simulink model "CCMDcMotor"  (R2022b) (continued)
== system CCMDcMotor/DcMotorIOCtrlService == (continued)
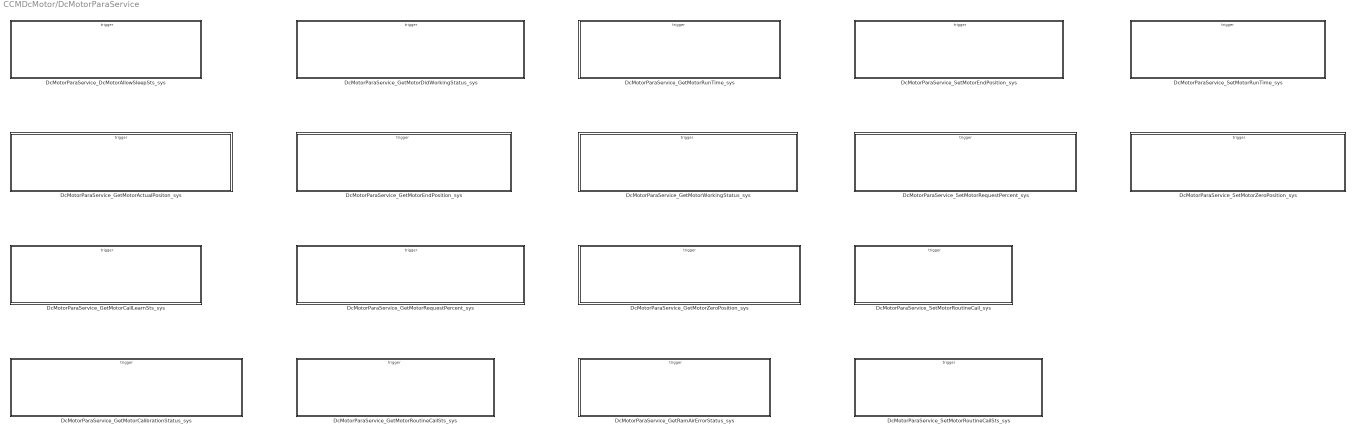
== system CCMDcMotor/DcMotorParaService ==
  DcMotorParaService_DcMotorAllowSleepSts_sys : Subsystem
  DcMotorParaService_GetMotorActualPositon_sys : Subsystem
  DcMotorParaService_GetMotorCailLearnSts_sys : Subsystem
  DcMotorParaService_GetMotorCalibrationStatus_sys : Subsystem
  DcMotorParaService_GetMotorDidWorkingStatus_sys : Subsystem
  DcMotorParaService_GetMotorEndPosition_sys : Subsystem
  DcMotorParaService_GetMotorRequestPercent_sys : Subsystem
  DcMotorParaService_GetMotorRoutineCailSts_sys : Subsystem
  DcMotorParaService_GetMotorRunTime_sys : Subsystem
  DcMotorParaService_GetMotorWorkingStatus_sys : Subsystem
  DcMotorParaService_GetMotorZeroPosition_sys : Subsystem
  DcMotorParaService_GetRamAirErrorStatus_sys : Subsystem
  DcMotorParaService_SetMotorEndPosition_sys : Subsystem
  DcMotorParaService_SetMotorRequestPercent_sys : Subsystem
  DcMotorParaService_SetMotorRoutineCailSts_sys : Subsystem
  DcMotorParaService_SetMotorRoutineCail_sys : Subsystem
  DcMotorParaService_SetMotorRunTime_sys : Subsystem
  DcMotorParaService_SetMotorZeroPosition_sys : Subsystem

== system CCMDcMotor/DcMtrCaliServive ==
  DcMtrCaliServive_SetAllCalibrate_sys : Subsystem
  DcMtrCaliServive_SetMotorCalibrate_sys : Subsystem

== system CCMDcMotor/DcMotNvMClrService/DcMotNvMClrService_ClearNvMData_sys ==
  function : TriggerPort  TriggerType=function-call  IsSimulinkFunction=on  FunctionName=DcMotNvMClrService_ClearNvMData  VariantControl=(inherit)
  Ground_1 : Ground
  PA_NvMBlockDcMtrCalDataInit : Constant  Value=NvMBlockDcMtrCalDataInit
  Terminator_1 : Terminator
  ERR : ArgOut  OutDataTypeStr=Std_ReturnType  ArgumentName=ERR
  wires:
  PA_NvMBlockDcMtrCalDataInit/1 -> Terminator_1/1
  Ground_1/1 -> ERR/1

== system CCMDcMotor/DcMotorDtcService/DcMotorDtcService_Motor0CtrOpenDtcStatus_sys ==
  function : TriggerPort  TriggerType=function-call  IsSimulinkFunction=on  FunctionName=DcMotorDtcService_Motor0CtrOpenDtcStatus  VariantControl=(inherit)
  Ground_1 : Ground
  Ground_2 : Ground
  PA_NvMBlockDcMtrCalDataInit : Constant  Value=NvMBlockDcMtrCalDataInit
  Terminator_1 : Terminator
  Status : ArgOut  OutDataTypeStr=Enum: DTCState  ArgumentName=Status
  ERR : ArgOut  Port=2  OutDataTypeStr=Std_ReturnType  ArgumentName=ERR
  wires:
  PA_NvMBlockDcMtrCalDataInit/1 -> Terminator_1/1
  Ground_1/1 -> Status/1
  Ground_2/1 -> ERR/1

== system CCMDcMotor/DcMotorDtcService/DcMotorDtcService_Motor0CtrShortBatDtcStatus_sys ==
  function : TriggerPort  TriggerType=function-call  IsSimulinkFunction=on  FunctionName=DcMotorDtcService_Motor0CtrShortBatDtcStatus  VariantControl=(inherit)
  Ground_1 : Ground
  Ground_2 : Ground
  PA_NvMBlockDcMtrCalDataInit : Constant  Value=NvMBlockDcMtrCalDataInit
  Terminator_1 : Terminator
  Status : ArgOut  OutDataTypeStr=Enum: DTCState  ArgumentName=Status
  ERR : ArgOut  Port=2  OutDataTypeStr=Std_ReturnType  ArgumentName=ERR
  wires:
  PA_NvMBlockDcMtrCalDataInit/1 -> Terminator_1/1
  Ground_1/1 -> Status/1
  Ground_2/1 -> ERR/1

== system CCMDcMotor/DcMotorDtcService/DcMotorDtcService_Motor0FbOpenDtcStatus_sys ==
  function : TriggerPort  TriggerType=function-call  IsSimulinkFunction=on  FunctionName=DcMotorDtcService_Motor0FbOpenDtcStatus  VariantControl=(inherit)
  Ground_1 : Ground
  Ground_2 : Ground
  PA_NvMBlockDcMtrCalDataInit : Constant  Value=NvMBlockDcMtrCalDataInit
  Terminator_1 : Terminator
  Status : ArgOut  OutDataTypeStr=Enum: DTCState  ArgumentName=Status
  ERR : ArgOut  Port=2  OutDataTypeStr=Std_ReturnType  ArgumentName=ERR
  wires:
  PA_NvMBlockDcMtrCalDataInit/1 -> Terminator_1/1
  Ground_1/1 -> Status/1
  Ground_2/1 -> ERR/1

== system CCMDcMotor/DcMotorDtcService/DcMotorDtcService_Motor0FbShortBatDtcStatus_sys ==
  function : TriggerPort  TriggerType=function-call  IsSimulinkFunction=on  FunctionName=DcMotorDtcService_Motor0FbShortBatDtcStatus  VariantControl=(inherit)
  Ground_1 : Ground
  Ground_2 : Ground
  PA_NvMBlockDcMtrCalDataInit : Constant  Value=NvMBlockDcMtrCalDataInit
  Terminator_1 : Terminator
  Status : ArgOut  OutDataTypeStr=Enum: DTCState  ArgumentName=Status
  ERR : ArgOut  Port=2  OutDataTypeStr=Std_ReturnType  ArgumentName=ERR
  wires:
  PA_NvMBlockDcMtrCalDataInit/1 -> Terminator_1/1
  Ground_1/1 -> Status/1
  Ground_2/1 -> ERR/1

== system CCMDcMotor/DcMotorDtcService/DcMotorDtcService_Motor0StallDtcStatus_sys ==
  function : TriggerPort  TriggerType=function-call  IsSimulinkFunction=on  FunctionName=DcMotorDtcService_Motor0StallDtcStatus  VariantControl=(inherit)
  Ground_1 : Ground
  Ground_2 : Ground
  PA_NvMBlockDcMtrCalDataInit : Constant  Value=NvMBlockDcMtrCalDataInit
  Terminator_1 : Terminator
  Status : ArgOut  OutDataTypeStr=Enum: DTCState  ArgumentName=Status
  ERR : ArgOut  Port=2  OutDataTypeStr=Std_ReturnType  ArgumentName=ERR
  wires:
  PA_NvMBlockDcMtrCalDataInit/1 -> Terminator_1/1
  Ground_1/1 -> Status/1
  Ground_2/1 -> ERR/1

== system CCMDcMotor/DcMotorDtcService/DcMotorDtcService_Motor1CtrOpenDtcStatus_sys ==
  function : TriggerPort  TriggerType=function-call  IsSimulinkFunction=on  FunctionName=DcMotorDtcService_Motor1CtrOpenDtcStatus  VariantControl=(inherit)
  Ground_1 : Ground
  Ground_2 : Ground
  PA_NvMBlockDcMtrCalDataInit : Constant  Value=NvMBlockDcMtrCalDataInit
  Terminator_1 : Terminator
  Status : ArgOut  OutDataTypeStr=Enum: DTCState  ArgumentName=Status
  ERR : ArgOut  Port=2  OutDataTypeStr=Std_ReturnType  ArgumentName=ERR
  wires:
  PA_NvMBlockDcMtrCalDataInit/1 -> Terminator_1/1
  Ground_1/1 -> Status/1
  Ground_2/1 -> ERR/1

== system CCMDcMotor/DcMotorDtcService/DcMotorDtcService_Motor1CtrShortBatDtcStatus_sys ==
  function : TriggerPort  TriggerType=function-call  IsSimulinkFunction=on  FunctionName=DcMotorDtcService_Motor1CtrShortBatDtcStatus  VariantControl=(inherit)
  Ground_1 : Ground
  Ground_2 : Ground
  PA_NvMBlockDcMtrCalDataInit : Constant  Value=NvMBlockDcMtrCalDataInit
  Terminator_1 : Terminator
  Status : ArgOut  OutDataTypeStr=Enum: DTCState  ArgumentName=Status
  ERR : ArgOut  Port=2  OutDataTypeStr=Std_ReturnType  ArgumentName=ERR
  wires:
  PA_NvMBlockDcMtrCalDataInit/1 -> Terminator_1/1
  Ground_1/1 -> Status/1
  Ground_2/1 -> ERR/1

== system CCMDcMotor/DcMotorDtcService/DcMotorDtcService_Motor1FbOpenDtcStatus_sys ==
  function : TriggerPort  TriggerType=function-call  IsSimulinkFunction=on  FunctionName=DcMotorDtcService_Motor1FbOpenDtcStatus  VariantControl=(inherit)
  Ground_1 : Ground
  Ground_2 : Ground
  PA_NvMBlockDcMtrCalDataInit : Constant  Value=NvMBlockDcMtrCalDataInit
  Terminator_1 : Terminator
  Status : ArgOut  OutDataTypeStr=Enum: DTCState  ArgumentName=Status
  ERR : ArgOut  Port=2  OutDataTypeStr=Std_ReturnType  ArgumentName=ERR
  wires:
  PA_NvMBlockDcMtrCalDataInit/1 -> Terminator_1/1
  Ground_1/1 -> Status/1
  Ground_2/1 -> ERR/1

== system CCMDcMotor/DcMotorDtcService/DcMotorDtcService_Motor1FbShortBatDtcStatus_sys ==
  function : TriggerPort  TriggerType=function-call  IsSimulinkFunction=on  FunctionName=DcMotorDtcService_Motor1FbShortBatDtcStatus  VariantControl=(inherit)
  Ground_1 : Ground
  Ground_2 : Ground
  PA_NvMBlockDcMtrCalDataInit : Constant  Value=NvMBlockDcMtrCalDataInit
  Terminator_1 : Terminator
  Status : ArgOut  OutDataTypeStr=Enum: DTCState  ArgumentName=Status
  ERR : ArgOut  Port=2  OutDataTypeStr=Std_ReturnType  ArgumentName=ERR
  wires:
  PA_NvMBlockDcMtrCalDataInit/1 -> Terminator_1/1
  Ground_1/1 -> Status/1
  Ground_2/1 -> ERR/1

== system CCMDcMotor/DcMotorDtcService/DcMotorDtcService_Motor1StallDtcStatus_sys ==
  function : TriggerPort  TriggerType=function-call  IsSimulinkFunction=on  FunctionName=DcMotorDtcService_Motor1StallDtcStatus  VariantControl=(inherit)
  Ground_1 : Ground
  Ground_2 : Ground
  PA_NvMBlockDcMtrCalDataInit : Constant  Value=NvMBlockDcMtrCalDataInit
  Terminator_1 : Terminator
  Status : ArgOut  OutDataTypeStr=Enum: DTCState  ArgumentName=Status
  ERR : ArgOut  Port=2  OutDataTypeStr=Std_ReturnType  ArgumentName=ERR
  wires:
  PA_NvMBlockDcMtrCalDataInit/1 -> Terminator_1/1
  Ground_1/1 -> Status/1
  Ground_2/1 -> ERR/1

== system CCMDcMotor/DcMotorDtcService/DcMotorDtcService_Motor2CtrOpenDtcStatus_sys ==
  function : TriggerPort  TriggerType=function-call  IsSimulinkFunction=on  FunctionName=DcMotorDtcService_Motor2CtrOpenDtcStatus  VariantControl=(inherit)
  Ground_1 : Ground
  Ground_2 : Ground
  PA_NvMBlockDcMtrCalDataInit : Constant  Value=NvMBlockDcMtrCalDataInit
  Terminator_1 : Terminator
  Status : ArgOut  OutDataTypeStr=Enum: DTCState  ArgumentName=Status
  ERR : ArgOut  Port=2  OutDataTypeStr=Std_ReturnType  ArgumentName=ERR
  wires:
  PA_NvMBlockDcMtrCalDataInit/1 -> Terminator_1/1
  Ground_1/1 -> Status/1
  Ground_2/1 -> ERR/1

== system CCMDcMotor/DcMotorDtcService/DcMotorDtcService_Motor2CtrShortBatDtcStatus_sys ==
  function : TriggerPort  TriggerType=function-call  IsSimulinkFunction=on  FunctionName=DcMotorDtcService_Motor2CtrShortBatDtcStatus  VariantControl=(inherit)
  Ground_1 : Ground
  Ground_2 : Ground
  PA_NvMBlockDcMtrCalDataInit : Constant  Value=NvMBlockDcMtrCalDataInit
  Terminator_1 : Terminator
  Status : ArgOut  OutDataTypeStr=Enum: DTCState  ArgumentName=Status
  ERR : ArgOut  Port=2  OutDataTypeStr=Std_ReturnType  ArgumentName=ERR
  wires:
  PA_NvMBlockDcMtrCalDataInit/1 -> Terminator_1/1
  Ground_1/1 -> Status/1
  Ground_2/1 -> ERR/1

== system CCMDcMotor/DcMotorDtcService/DcMotorDtcService_Motor2FbOpenDtcStatus_sys ==
  function : TriggerPort  TriggerType=function-call  IsSimulinkFunction=on  FunctionName=DcMotorDtcService_Motor2FbOpenDtcStatus  VariantControl=(inherit)
  Ground_1 : Ground
  Ground_2 : Ground
  PA_NvMBlockDcMtrCalDataInit : Constant  Value=NvMBlockDcMtrCalDataInit
  Terminator_1 : Terminator
  Status : ArgOut  OutDataTypeStr=Enum: DTCState  ArgumentName=Status
  ERR : ArgOut  Port=2  OutDataTypeStr=Std_ReturnType  ArgumentName=ERR
  wires:
  PA_NvMBlockDcMtrCalDataInit/1 -> Terminator_1/1
  Ground_1/1 -> Status/1
  Ground_2/1 -> ERR/1

== system CCMDcMotor/DcMotorDtcService/DcMotorDtcService_Motor2FbShortBatDtcStatus_sys ==
  function : TriggerPort  TriggerType=function-call  IsSimulinkFunction=on  FunctionName=DcMotorDtcService_Motor2FbShortBatDtcStatus  VariantControl=(inherit)
  Ground_1 : Ground
  Ground_2 : Ground
  PA_NvMBlockDcMtrCalDataInit : Constant  Value=NvMBlockDcMtrCalDataInit
  Terminator_1 : Terminator
  Status : ArgOut  OutDataTypeStr=Enum: DTCState  ArgumentName=Status
  ERR : ArgOut  Port=2  OutDataTypeStr=Std_ReturnType  ArgumentName=ERR
  wires:
  PA_NvMBlockDcMtrCalDataInit/1 -> Terminator_1/1
  Ground_1/1 -> Status/1
  Ground_2/1 -> ERR/1

== system CCMDcMotor/DcMotorDtcService/DcMotorDtcService_Motor2StallDtcStatus_sys ==
  function : TriggerPort  TriggerType=function-call  IsSimulinkFunction=on  FunctionName=DcMotorDtcService_Motor2StallDtcStatus  VariantControl=(inherit)
  Ground_1 : Ground
  Ground_2 : Ground
  PA_NvMBlockDcMtrCalDataInit : Constant  Value=NvMBlockDcMtrCalDataInit
  Terminator_1 : Terminator
  Status : ArgOut  OutDataTypeStr=Enum: DTCState  ArgumentName=Status
  ERR : ArgOut  Port=2  OutDataTypeStr=Std_ReturnType  ArgumentName=ERR
  wires:
  PA_NvMBlockDcMtrCalDataInit/1 -> Terminator_1/1
  Ground_1/1 -> Status/1
  Ground_2/1 -> ERR/1

== system CCMDcMotor/DcMotorDtcService/DcMotorDtcService_Motor3CtrOpenDtcStatus_sys ==
  function : TriggerPort  TriggerType=function-call  IsSimulinkFunction=on  FunctionName=DcMotorDtcService_Motor3CtrOpenDtcStatus  VariantControl=(inherit)
  Ground_1 : Ground
  Ground_2 : Ground
  PA_NvMBlockDcMtrCalDataInit : Constant  Value=NvMBlockDcMtrCalDataInit
  Terminator_1 : Terminator
  Status : ArgOut  OutDataTypeStr=Enum: DTCState  ArgumentName=Status
  ERR : ArgOut  Port=2  OutDataTypeStr=Std_ReturnType  ArgumentName=ERR
  wires:
  PA_NvMBlockDcMtrCalDataInit/1 -> Terminator_1/1
  Ground_1/1 -> Status/1
  Ground_2/1 -> ERR/1

== system CCMDcMotor/DcMotorDtcService/DcMotorDtcService_Motor3CtrShortBatDtcStatus_sys ==
  function : TriggerPort  TriggerType=function-call  IsSimulinkFunction=on  FunctionName=DcMotorDtcService_Motor3CtrShortBatDtcStatus  VariantControl=(inherit)
  Ground_1 : Ground
  Ground_2 : Ground
  PA_NvMBlockDcMtrCalDataInit : Constant  Value=NvMBlockDcMtrCalDataInit
  Terminator_1 : Terminator
  Status : ArgOut  OutDataTypeStr=Enum: DTCState  ArgumentName=Status
  ERR : ArgOut  Port=2  OutDataTypeStr=Std_ReturnType  ArgumentName=ERR
  wires:
  PA_NvMBlockDcMtrCalDataInit/1 -> Terminator_1/1
  Ground_1/1 -> Status/1
  Ground_2/1 -> ERR/1

== system CCMDcMotor/DcMotorDtcService/DcMotorDtcService_Motor3FbOpenDtcStatus_sys ==
  function : TriggerPort  TriggerType=function-call  IsSimulinkFunction=on  FunctionName=DcMotorDtcService_Motor3FbOpenDtcStatus  VariantControl=(inherit)
  Ground_1 : Ground
  Ground_2 : Ground
  PA_NvMBlockDcMtrCalDataInit : Constant  Value=NvMBlockDcMtrCalDataInit
  Terminator_1 : Terminator
  Status : ArgOut  OutDataTypeStr=Enum: DTCState  ArgumentName=Status
  ERR : ArgOut  Port=2  OutDataTypeStr=Std_ReturnType  ArgumentName=ERR
  wires:
  PA_NvMBlockDcMtrCalDataInit/1 -> Terminator_1/1
  Ground_1/1 -> Status/1
  Ground_2/1 -> ERR/1

== system CCMDcMotor/DcMotorDtcService/DcMotorDtcService_Motor3FbShortBatDtcStatus_sys ==
  function : TriggerPort  TriggerType=function-call  IsSimulinkFunction=on  FunctionName=DcMotorDtcService_Motor3FbShortBatDtcStatus  VariantControl=(inherit)
  Ground_1 : Ground
  Ground_2 : Ground
  PA_NvMBlockDcMtrCalDataInit : Constant  Value=NvMBlockDcMtrCalDataInit
  Terminator_1 : Terminator
  Status : ArgOut  OutDataTypeStr=Enum: DTCState  ArgumentName=Status
  ERR : ArgOut  Port=2  OutDataTypeStr=Std_ReturnType  ArgumentName=ERR
  wires:
  PA_NvMBlockDcMtrCalDataInit/1 -> Terminator_1/1
  Ground_1/1 -> Status/1
  Ground_2/1 -> ERR/1

== system CCMDcMotor/DcMotorDtcService/DcMotorDtcService_Motor3StallDtcStatus_sys ==
  function : TriggerPort  TriggerType=function-call  IsSimulinkFunction=on  FunctionName=DcMotorDtcService_Motor3StallDtcStatus  VariantControl=(inherit)
  Ground_1 : Ground
  Ground_2 : Ground
  PA_NvMBlockDcMtrCalDataInit : Constant  Value=NvMBlockDcMtrCalDataInit
  Terminator_1 : Terminator
  Status : ArgOut  OutDataTypeStr=Enum: DTCState  ArgumentName=Status
  ERR : ArgOut  Port=2  OutDataTypeStr=Std_ReturnType  ArgumentName=ERR
  wires:
  PA_NvMBlockDcMtrCalDataInit/1 -> Terminator_1/1
  Ground_1/1 -> Status/1
  Ground_2/1 -> ERR/1

== system CCMDcMotor/DcMotorDtcService/DcMotorDtcService_Motor4CtrOpenDtcStatus_sys ==
  function : TriggerPort  TriggerType=function-call  IsSimulinkFunction=on  FunctionName=DcMotorDtcService_Motor4CtrOpenDtcStatus  VariantControl=(inherit)
  Ground_1 : Ground
  Ground_2 : Ground
  PA_NvMBlockDcMtrCalDataInit : Constant  Value=NvMBlockDcMtrCalDataInit
  Terminator_1 : Terminator
  Status : ArgOut  OutDataTypeStr=Enum: DTCState  ArgumentName=Status
  ERR : ArgOut  Port=2  OutDataTypeStr=Std_ReturnType  ArgumentName=ERR
  wires:
  PA_NvMBlockDcMtrCalDataInit/1 -> Terminator_1/1
  Ground_1/1 -> Status/1
  Ground_2/1 -> ERR/1

== system CCMDcMotor/DcMotorDtcService/DcMotorDtcService_Motor4CtrShortBatDtcStatus_sys ==
  function : TriggerPort  TriggerType=function-call  IsSimulinkFunction=on  FunctionName=DcMotorDtcService_Motor4CtrShortBatDtcStatus  VariantControl=(inherit)
  Ground_1 : Ground
  Ground_2 : Ground
  PA_NvMBlockDcMtrCalDataInit : Constant  Value=NvMBlockDcMtrCalDataInit
  Terminator_1 : Terminator
  Status : ArgOut  OutDataTypeStr=Enum: DTCState  ArgumentName=Status
  ERR : ArgOut  Port=2  OutDataTypeStr=Std_ReturnType  ArgumentName=ERR
  wires:
  PA_NvMBlockDcMtrCalDataInit/1 -> Terminator_1/1
  Ground_1/1 -> Status/1
  Ground_2/1 -> ERR/1

== system CCMDcMotor/DcMotorDtcService/DcMotorDtcService_Motor4FbOpenDtcStatus_sys ==
  function : TriggerPort  TriggerType=function-call  IsSimulinkFunction=on  FunctionName=DcMotorDtcService_Motor4FbOpenDtcStatus  VariantControl=(inherit)
  Ground_1 : Ground
  Ground_2 : Ground
  PA_NvMBlockDcMtrCalDataInit : Constant  Value=NvMBlockDcMtrCalDataInit
  Terminator_1 : Terminator
  Status : ArgOut  OutDataTypeStr=Enum: DTCState  ArgumentName=Status
  ERR : ArgOut  Port=2  OutDataTypeStr=Std_ReturnType  ArgumentName=ERR
  wires:
  PA_NvMBlockDcMtrCalDataInit/1 -> Terminator_1/1
  Ground_1/1 -> Status/1
  Ground_2/1 -> ERR/1

== system CCMDcMotor/DcMotorDtcService/DcMotorDtcService_Motor4FbShortBatDtcStatus_sys ==
  function : TriggerPort  TriggerType=function-call  IsSimulinkFunction=on  FunctionName=DcMotorDtcService_Motor4FbShortBatDtcStatus  VariantControl=(inherit)
  Ground_1 : Ground
  Ground_2 : Ground
  PA_NvMBlockDcMtrCalDataInit : Constant  Value=NvMBlockDcMtrCalDataInit
  Terminator_1 : Terminator
  Status : ArgOut  OutDataTypeStr=Enum: DTCState  ArgumentName=Status
  ERR : ArgOut  Port=2  OutDataTypeStr=Std_ReturnType  ArgumentName=ERR
  wires:
  PA_NvMBlockDcMtrCalDataInit/1 -> Terminator_1/1
  Ground_1/1 -> Status/1
  Ground_2/1 -> ERR/1

== system CCMDcMotor/DcMotorDtcService/DcMotorDtcService_Motor4StallDtcStatus_sys ==
  function : TriggerPort  TriggerType=function-call  IsSimulinkFunction=on  FunctionName=DcMotorDtcService_Motor4StallDtcStatus  VariantControl=(inherit)
  Ground_1 : Ground
  Ground_2 : Ground
  PA_NvMBlockDcMtrCalDataInit : Constant  Value=NvMBlockDcMtrCalDataInit
  Terminator_1 : Terminator
  Status : ArgOut  OutDataTypeStr=Enum: DTCState  ArgumentName=Status
  ERR : ArgOut  Port=2  OutDataTypeStr=Std_ReturnType  ArgumentName=ERR
  wires:
  PA_NvMBlockDcMtrCalDataInit/1 -> Terminator_1/1
  Ground_1/1 -> Status/1
  Ground_2/1 -> ERR/1

== system CCMDcMotor/DcMotorDtcService/DcMotorDtcService_Motor5CtrOpenDtcStatus_sys ==
  function : TriggerPort  TriggerType=function-call  IsSimulinkFunction=on  FunctionName=DcMotorDtcService_Motor5CtrOpenDtcStatus  VariantControl=(inherit)
  Ground_1 : Ground
  Ground_2 : Ground
  PA_NvMBlockDcMtrCalDataInit : Constant  Value=NvMBlockDcMtrCalDataInit
  Terminator_1 : Terminator
  Status : ArgOut  OutDataTypeStr=Enum: DTCState  ArgumentName=Status
  ERR : ArgOut  Port=2  OutDataTypeStr=Std_ReturnType  ArgumentName=ERR
  wires:
  PA_NvMBlockDcMtrCalDataInit/1 -> Terminator_1/1
  Ground_1/1 -> Status/1
  Ground_2/1 -> ERR/1

== system CCMDcMotor/DcMotorDtcService/DcMotorDtcService_Motor5CtrShortBatDtcStatus_sys ==
  function : TriggerPort  TriggerType=function-call  IsSimulinkFunction=on  FunctionName=DcMotorDtcService_Motor5CtrShortBatDtcStatus  VariantControl=(inherit)
  Ground_1 : Ground
  Ground_2 : Ground
  PA_NvMBlockDcMtrCalDataInit : Constant  Value=NvMBlockDcMtrCalDataInit
  Terminator_1 : Terminator
  Status : ArgOut  OutDataTypeStr=Enum: DTCState  ArgumentName=Status
  ERR : ArgOut  Port=2  OutDataTypeStr=Std_ReturnType  ArgumentName=ERR
  wires:
  PA_NvMBlockDcMtrCalDataInit/1 -> Terminator_1/1
  Ground_1/1 -> Status/1
  Ground_2/1 -> ERR/1

== system CCMDcMotor/DcMotorDtcService/DcMotorDtcService_Motor5FbOpenDtcStatus_sys ==
  function : TriggerPort  TriggerType=function-call  IsSimulinkFunction=on  FunctionName=DcMotorDtcService_Motor5FbOpenDtcStatus  VariantControl=(inherit)
  Ground_1 : Ground
  Ground_2 : Ground
  PA_NvMBlockDcMtrCalDataInit : Constant  Value=NvMBlockDcMtrCalDataInit
  Terminator_1 : Terminator
  Status : ArgOut  OutDataTypeStr=Enum: DTCState  ArgumentName=Status
  ERR : ArgOut  Port=2  OutDataTypeStr=Std_ReturnType  ArgumentName=ERR
  wires:
  PA_NvMBlockDcMtrCalDataInit/1 -> Terminator_1/1
  Ground_1/1 -> Status/1
  Ground_2/1 -> ERR/1

== system CCMDcMotor/DcMotorDtcService/DcMotorDtcService_Motor5FbShortBatDtcStatus_sys ==
  function : TriggerPort  TriggerType=function-call  IsSimulinkFunction=on  FunctionName=DcMotorDtcService_Motor5FbShortBatDtcStatus  VariantControl=(inherit)
  Ground_1 : Ground
  Ground_2 : Ground
  PA_NvMBlockDcMtrCalDataInit : Constant  Value=NvMBlockDcMtrCalDataInit
  Terminator_1 : Terminator
  Status : ArgOut  OutDataTypeStr=Enum: DTCState  ArgumentName=Status
  ERR : ArgOut  Port=2  OutDataTypeStr=Std_ReturnType  ArgumentName=ERR
  wires:
  PA_NvMBlockDcMtrCalDataInit/1 -> Terminator_1/1
  Ground_1/1 -> Status/1
  Ground_2/1 -> ERR/1

== system CCMDcMotor/DcMotorDtcService/DcMotorDtcService_Motor5StallDtcStatus_sys ==
  function : TriggerPort  TriggerType=function-call  IsSimulinkFunction=on  FunctionName=DcMotorDtcService_Motor5StallDtcStatus  VariantControl=(inherit)
  Ground_1 : Ground
  Ground_2 : Ground
  PA_NvMBlockDcMtrCalDataInit : Constant  Value=NvMBlockDcMtrCalDataInit
  Terminator_1 : Terminator
  Status : ArgOut  OutDataTypeStr=Enum: DTCState  ArgumentName=Status
  ERR : ArgOut  Port=2  OutDataTypeStr=Std_ReturnType  ArgumentName=ERR
  wires:
  PA_NvMBlockDcMtrCalDataInit/1 -> Terminator_1/1
  Ground_1/1 -> Status/1
  Ground_2/1 -> ERR/1

== system CCMDcMotor/DcMotorDtcService/DcMotorDtcService_Motor6CtrOpenDtcStatus_sys ==
  function : TriggerPort  TriggerType=function-call  IsSimulinkFunction=on  FunctionName=DcMotorDtcService_Motor6CtrOpenDtcStatus  VariantControl=(inherit)
  Ground_1 : Ground
  Ground_2 : Ground
  PA_NvMBlockDcMtrCalDataInit : Constant  Value=NvMBlockDcMtrCalDataInit
  Terminator_1 : Terminator
  Status : ArgOut  OutDataTypeStr=Enum: DTCState  ArgumentName=Status
  ERR : ArgOut  Port=2  OutDataTypeStr=Std_ReturnType  ArgumentName=ERR
  wires:
  PA_NvMBlockDcMtrCalDataInit/1 -> Terminator_1/1
  Ground_1/1 -> Status/1
  Ground_2/1 -> ERR/1

== system CCMDcMotor/DcMotorDtcService/DcMotorDtcService_Motor6CtrShortBatDtcStatus_sys ==
  function : TriggerPort  TriggerType=function-call  IsSimulinkFunction=on  FunctionName=DcMotorDtcService_Motor6CtrShortBatDtcStatus  VariantControl=(inherit)
  Ground_1 : Ground
  Ground_2 : Ground
  PA_NvMBlockDcMtrCalDataInit : Constant  Value=NvMBlockDcMtrCalDataInit
  Terminator_1 : Terminator
  Status : ArgOut  OutDataTypeStr=Enum: DTCState  ArgumentName=Status
  ERR : ArgOut  Port=2  OutDataTypeStr=Std_ReturnType  ArgumentName=ERR
  wires:
  PA_NvMBlockDcMtrCalDataInit/1 -> Terminator_1/1
  Ground_1/1 -> Status/1
  Ground_2/1 -> ERR/1

== system CCMDcMotor/DcMotorDtcService/DcMotorDtcService_Motor6FbOpenDtcStatus_sys ==
  function : TriggerPort  TriggerType=function-call  IsSimulinkFunction=on  FunctionName=DcMotorDtcService_Motor6FbOpenDtcStatus  VariantControl=(inherit)
  Ground_1 : Ground
  Ground_2 : Ground
  PA_NvMBlockDcMtrCalDataInit : Constant  Value=NvMBlockDcMtrCalDataInit
  Terminator_1 : Terminator
  Status : ArgOut  OutDataTypeStr=Enum: DTCState  ArgumentName=Status
  ERR : ArgOut  Port=2  OutDataTypeStr=Std_ReturnType  ArgumentName=ERR
  wires:
  PA_NvMBlockDcMtrCalDataInit/1 -> Terminator_1/1
  Ground_1/1 -> Status/1
  Ground_2/1 -> ERR/1

== system CCMDcMotor/DcMotorDtcService/DcMotorDtcService_Motor6FbShortBatDtcStatus_sys ==
  function : TriggerPort  TriggerType=function-call  IsSimulinkFunction=on  FunctionName=DcMotorDtcService_Motor6FbShortBatDtcStatus  VariantControl=(inherit)
  Ground_1 : Ground
  Ground_2 : Ground
  PA_NvMBlockDcMtrCalDataInit : Constant  Value=NvMBlockDcMtrCalDataInit
  Terminator_1 : Terminator
  Status : ArgOut  OutDataTypeStr=Enum: DTCState  ArgumentName=Status
  ERR : ArgOut  Port=2  OutDataTypeStr=Std_ReturnType  ArgumentName=ERR
  wires:
  PA_NvMBlockDcMtrCalDataInit/1 -> Terminator_1/1
  Ground_1/1 -> Status/1
  Ground_2/1 -> ERR/1

== system CCMDcMotor/DcMotorDtcService/DcMotorDtcService_Motor6StallDtcStatus_sys ==
  function : TriggerPort  TriggerType=function-call  IsSimulinkFunction=on  FunctionName=DcMotorDtcService_Motor6StallDtcStatus  VariantControl=(inherit)
  Ground_1 : Ground
  Ground_2 : Ground
  PA_NvMBlockDcMtrCalDataInit : Constant  Value=NvMBlockDcMtrCalDataInit
  Terminator_1 : Terminator
  Status : ArgOut  OutDataTypeStr=Enum: DTCState  ArgumentName=Status
  ERR : ArgOut  Port=2  OutDataTypeStr=Std_ReturnType  ArgumentName=ERR
  wires:
  PA_NvMBlockDcMtrCalDataInit/1 -> Terminator_1/1
  Ground_1/1 -> Status/1
  Ground_2/1 -> ERR/1

== system CCMDcMotor/DcMotorIOCtrlService/DcMotorIOCtrlService_FreezeMotorCurrentPosition_sys ==
  function : TriggerPort  TriggerType=function-call  IsSimulinkFunction=on  FunctionName=DcMotorIOCtrlService_FreezeMotorCurrentPosition  VariantControl=(inherit)
  Ground_1 : Ground
  PA_NvMBlockDcMtrCalDataInit : Constant  Value=NvMBlockDcMtrCalDataInit
  Terminator_1 : Terminator
  Terminator_2 : Terminator
  Channel : ArgIn  OutDataTypeStr=Enum: DcChlType  ArgumentName=Channel
  ERR : ArgOut  OutDataTypeStr=Std_ReturnType  ArgumentName=ERR
  wires:
  PA_NvMBlockDcMtrCalDataInit/1 -> Terminator_1/1
  Channel/1 -> Terminator_2/1
  Ground_1/1 -> ERR/1

== system CCMDcMotor/DcMotorIOCtrlService/DcMotorIOCtrlService_MotorRetCtlToECU_sys ==
  function : TriggerPort  TriggerType=function-call  IsSimulinkFunction=on  FunctionName=DcMotorIOCtrlService_MotorRetCtlToECU  VariantControl=(inherit)
  Ground_1 : Ground
  PA_NvMBlockDcMtrCalDataInit : Constant  Value=NvMBlockDcMtrCalDataInit
  Terminator_1 : Terminator
  Terminator_2 : Terminator
  Channel : ArgIn  OutDataTypeStr=Enum: DcChlType  ArgumentName=Channel
  ERR : ArgOut  OutDataTypeStr=Std_ReturnType  ArgumentName=ERR
  wires:
  PA_NvMBlockDcMtrCalDataInit/1 -> Terminator_1/1
  Channel/1 -> Terminator_2/1
  Ground_1/1 -> ERR/1

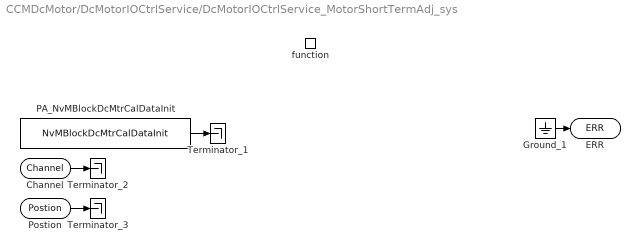
== system CCMDcMotor/DcMotorIOCtrlService/DcMotorIOCtrlService_MotorShortTermAdj_sys ==
  function : TriggerPort  TriggerType=function-call  IsSimulinkFunction=on  FunctionName=DcMotorIOCtrlService_MotorShortTermAdj  VariantControl=(inherit)
  Ground_1 : Ground
  PA_NvMBlockDcMtrCalDataInit : Constant  Value=NvMBlockDcMtrCalDataInit
  Terminator_1 : Terminator
  Terminator_2 : Terminator
  Terminator_3 : Terminator
  Channel : ArgIn  OutDataTypeStr=Enum: DcChlType  ArgumentName=Channel
  Postion : ArgIn  Port=2  OutDataTypeStr=UInt16  ArgumentName=Postion
  ERR : ArgOut  OutDataTypeStr=Std_ReturnType  ArgumentName=ERR
  wires:
  PA_NvMBlockDcMtrCalDataInit/1 -> Terminator_1/1
  Channel/1 -> Terminator_2/1
  Postion/1 -> Terminator_3/1
  Ground_1/1 -> ERR/1

== system CCMDcMotor/DcMotorParaService/DcMotorParaService_DcMotorAllowSleepSts_sys ==
  function : TriggerPort  TriggerType=function-call  IsSimulinkFunction=on  FunctionName=DcMotorParaService_DcMotorAllowSleepSts  VariantControl=(inherit)
  Ground_1 : Ground
  Ground_2 : Ground
  PA_NvMBlockDcMtrCalDataInit : Constant  Value=NvMBlockDcMtrCalDataInit
  Terminator_1 : Terminator
  Status : ArgOut  OutDataTypeStr=UInt8  ArgumentName=Status
  ERR : ArgOut  Port=2  OutDataTypeStr=Std_ReturnType  ArgumentName=ERR
  wires:
  PA_NvMBlockDcMtrCalDataInit/1 -> Terminator_1/1
  Ground_1/1 -> Status/1
  Ground_2/1 -> ERR/1
